simulink model "non_linear_final"  (R2021b)
settings: Solver=ode23  StartTime=0.0  StopTime=30  RelTol=1e-3
values from neighbouring files (symbols this model uses):
  A_ratio = 1.41    [non_linear_param_final.m]
  Annulus_area = Bore_area-Rod_area    (= 2356.19449019)    [non_linear_param_final.m]
  beta_F = 12000e5    (= 1200000000)    [non_linear_param_final.m]
  Bore_area = pi/4*Bore_diameter^2    (= 3318.30724035)    [non_linear_param_final.m]
  cyl_c_end = 9e8    (= 900000000)    [non_linear_param_final.m]
  cyl_c_visc = 1500    [non_linear_param_final.m]
  cyl_CH_stat = 1/50    (= 0.02)    [non_linear_param_final.m]
  cyl_F_coul = 75    [non_linear_param_final.m]
  cyl_FH_stat = 500    [non_linear_param_final.m]
  cyl_gamma = 250    [non_linear_param_final.m]
  cyl_k_end = 6e9    (= 6000000000)    [non_linear_param_final.m]
  DB = -0.114    [non_linear_param_final.m]
  dr_dcv = 0.7    [non_linear_param_final.m]
  Eff_Bulk_On = 1    [non_linear_param_final.m]
  eta_air = 0.7e-2    (= 0.007)    [non_linear_param_final.m]
  k_cv = 15    [non_linear_param_final.m]
  k_DCV_PA = 7.9    [non_linear_param_final.m]
  k_DCV_PB = 7.78    [non_linear_param_final.m]
  k_DCV_T = 7.9    [non_linear_param_final.m]
  k_ocv = 15    [non_linear_param_final.m]
  K_oil = 1.5e4*0+12000    (= 12000)    [non_linear_param_final.m]
  k_tanh = 0.005    [non_linear_param_final.m]
  kappa_air = 1.4    [non_linear_param_final.m]
  LargeValveOpening = 1    [non_linear_param_final.m]
  mu_ocv = 3    [non_linear_param_final.m]
  OL_pa = -0.114    [non_linear_param_final.m]
  OL_pb = 0.114    [non_linear_param_final.m]
  p0_A = 62.79    [non_linear_param_final.m]
  p0_A1 = 1.08    [non_linear_param_final.m]
  p0_B = 1.213    [non_linear_param_final.m]
  p_cv_fo = 1    [non_linear_param_final.m]
  p_cv_set = 2.8    [non_linear_param_final.m]
  p_ocv_fo = 350    [non_linear_param_final.m]
  p_ocv_set = 150    [non_linear_param_final.m]
  p_pc_set = 7    [non_linear_param_final.m]
  p_R = 1.1    [non_linear_param_final.m]
  p_S = 180    [non_linear_param_final.m]
  patm = 1.013e5    (= 101300)    [non_linear_param_final.m]
  Stroke = 500    [non_linear_param_final.m]
  t0 = 1    [non_linear_param_final.m]
  tau_ocv = 0.0889*0+0.055    (= 0.055)    [non_linear_param_final.m]
  tau_pc = 0.0064*0+0.009    (= 0.009)    [non_linear_param_final.m]
  tStepDown = 4.7*0+4.6    (= 4.6)    [non_linear_param_final.m]
  tStepUp = 6.2    [non_linear_param_final.m]
  tWait = 8.0    (= 8)    [non_linear_param_final.m]
  UL_at = -0.05    [non_linear_param_final.m]
  UL_bt = 0.05    [non_linear_param_final.m]
  Up = 1    [non_linear_param_final.m]
  uS_sp = -0.55    [non_linear_param_final.m]
  V_CH_A = 1.0e-03    (= 0.001)    [non_linear_param_final.m]
  V_CH_A1 = 0.1e-03    (= 0.0001)    [non_linear_param_final.m]
  V_CH_B = 1.25e-03    (= 0.00125)    [non_linear_param_final.m]
  v_cyl_0 = 0    [non_linear_param_final.m]
  wn_dcv = 30    [non_linear_param_final.m]
  x_cyl_0 = 0.45012    [non_linear_param_final.m]
undefined symbols (not defined in the file or in its folder): sigbuilder_block, uS0
libraries: eeGeneralControl x3
(part 1 of 2)
settings Model: Creator=Daniel Hagen  LastModifiedBy=danielh
settings SolverCC: ZeroCrossAlgorithm=Adaptive  AlgebraicLoopSolver=TrustRegion
settings DataIOCC: ReturnWorkspaceOutputs=off
settings DebuggingCC: IgnoredZcDiagnostic=none  SFMachineParentedDataDiag=warning  ArithmeticOperatorsInVariantConditions=warning
settings SFSimCC: CustomCodeUndefinedFunction=UseInterfaceOnly

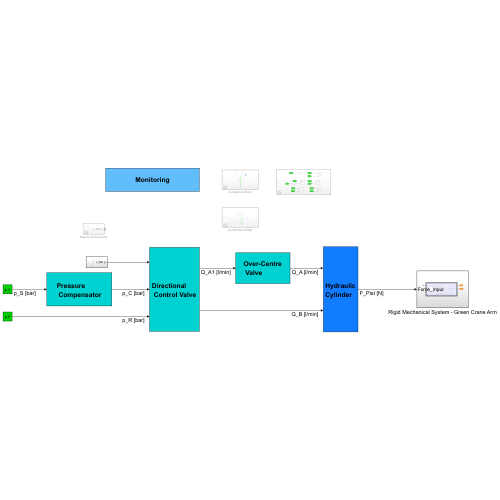
[thumbnail: Simulink's stored preview of the top-level diagram]
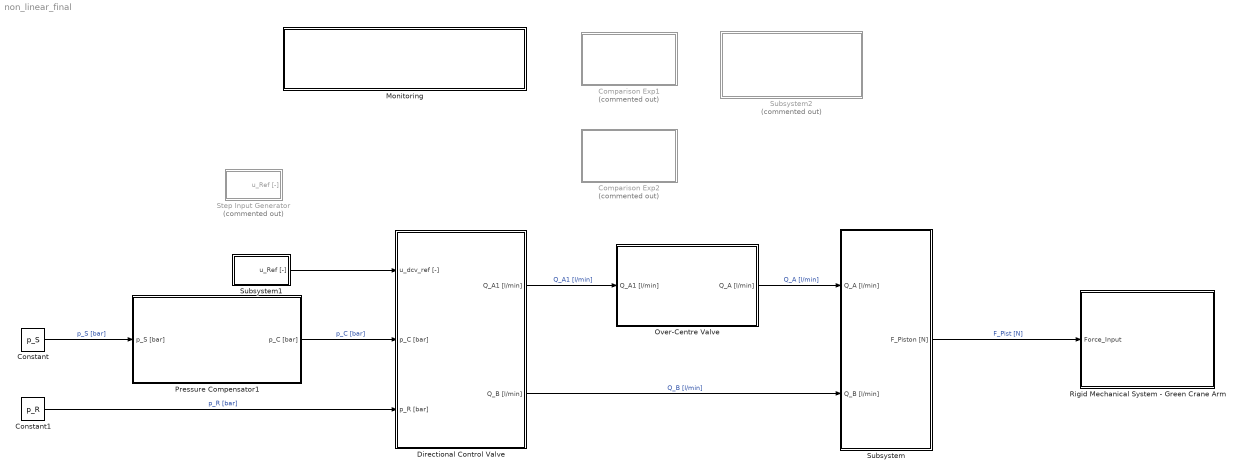
== system non_linear_final ==
  Comparison Exp1 : Subsystem  Commented=on
  Comparison Exp2 : Subsystem  Commented=on
  Constant : Constant  Value=p_S
  Constant1 : Constant  Value=p_R
  Directional Control Valve : Subsystem
      mask Display: disp('{\bf\fontsize{22}Directional\newline Control Valve}', 'texmode','on');
  Monitoring : Subsystem
      mask Display: disp('{\bf\fontsize{22}Monitoring}', 'texmode','on');
  Over-Centre Valve : Subsystem
      mask Display: disp('{\bf\fontsize{22}Over-Centre\newline Valve}', 'texmode','on');
  Pressure Compensator1 : Subsystem
      mask Display: disp('{\bf\fontsize{22}Pressure\newline Compensator}', 'texmode','on');
  Rigid Mechanical System - Green Crane Arm : Subsystem  VariantControl=Rigid_model
  Step Input Generator : Subsystem  Commented=on
  Subsystem : Subsystem
      mask Display: disp('{\bf\fontsize{22} Hydraulic \newline Cylinder}', 'texmode','on');
  Subsystem1 : Subsystem
  Subsystem2 : Subsystem  Commented=on
  wires:
  Directional Control Valve/1(Q_A1 [l/min]) -> Over-Centre Valve/1(Q_A1 [l/min])  "Q_A1 [l/min]"
  Subsystem/1(F_Piston [N]) -> Rigid Mechanical System - Green Crane Arm/1(Force_Input)  "F_Pist [N]"
  Pressure Compensator1/1(p_C [bar]) -> Directional Control Valve/2(p_C [bar])  "p_C [bar]"
  Constant/1 -> Pressure Compensator1/1(p_S [bar])  "p_S [bar]"
  Over-Centre Valve/1(Q_A [l/min]) -> Subsystem/1(Q_A [l/min])  "Q_A [l/min]"
  Constant1/1 -> Directional Control Valve/3(p_R [bar])  "p_R [bar]"
  Directional Control Valve/2(Q_B [l/min]) -> Subsystem/2(Q_B [l/min])  "Q_B [l/min]"
  Subsystem1/1(u_Ref [-]) -> Directional Control Valve/1(u_dcv_ref [-])

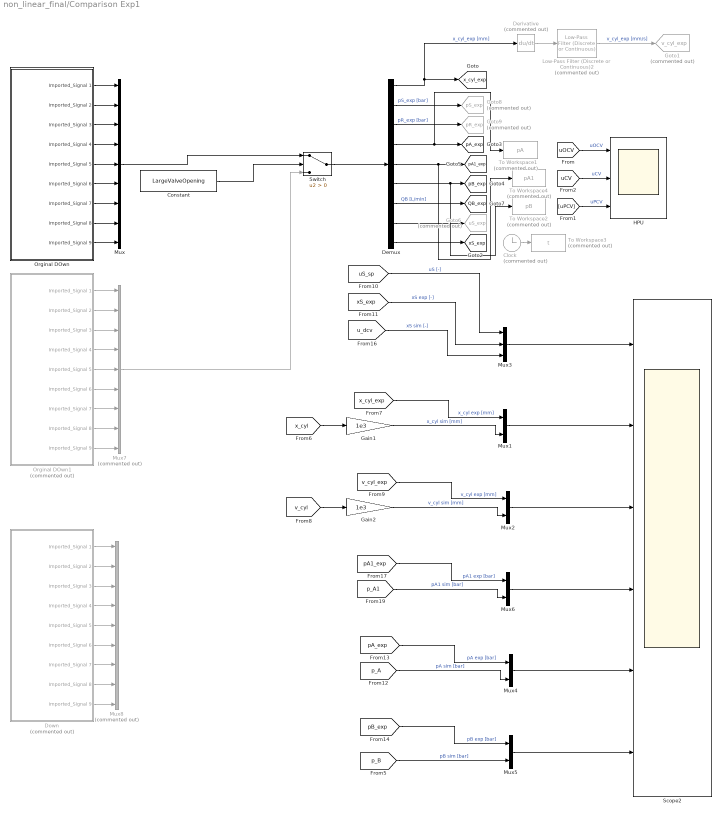
== system non_linear_final/Comparison Exp1 ==
  Clock : Clock  Commented=on
  Constant : Constant  Value=LargeValveOpening
  Demux : Demux  Outputs=9
  Derivative : Derivative  Commented=on
  Down : Subsystem  Tag=STV Subsys  Commented=on  LoadFcn=sigbuilder_block('load');  StartFcn=sigbuilder_block('start');  StopFcn=sigbuilder_block('stop');  ClipboardFcn=sigbuilder_block('clipboard');  mask(Sigbuilder block){tuvar=[0:10]}
      mask help: Simulink:masks:SigbuilderBlock_MD
      mask init:
      | if ~strcmp(get_param(bdroot(gcbh),'SimulationStatus'),'stopped') tuvar = sigbuilder_block('maskInit'); end
      settings: CopyFcn=sigbuilder_block('copy');  DeleteFcn=sigbuilder_block('delete');  ModelCloseFcn=sigbuilder_block('modelClose');  PreSaveFcn=sigbuilder_block('preSave');  PauseFcn=sigbuilder_block('pause');  ContinueFcn=sigbuilder_block('continue');  NameChangeFcn=sigbuilder_block('namechange');  OpenFcn=sigbuilder_block('open',[41.25 0 1392.75 801.75 ]);  CloseFcn=sigbuilder_block('close');
      mask Display: plot(0, 0, 100, 100,[2, 2, 32, 32, 2], [68, 8, 8, 68, 68],[32, 2], [38, 38], [32, 19, 2],[53, 60, 44], [32, 17, 17, 2],[16, 16, 31, 31]);txt = getActiveGroup(gcbh);text(2, 100, txt,'verticalAlignment', 'top');
  Gain1 : Gain  Gain=1e3
  Gain2 : Gain  Gain=1e3
  Goto1 : Goto  Commented=on  GotoTag=v_cyl_exp  TagVisibility=global
  Goto6 : Goto  Commented=on  GotoTag=uS_exp  TagVisibility=global
  Goto8 : Goto  Commented=on  GotoTag=pS_exp  TagVisibility=global
  Goto9 : Goto  Commented=on  GotoTag=pR_exp  TagVisibility=global
  HPU : Scope  NumInputPorts=3
  Low-Pass Filter (Discrete or Continuous)2 : eeGeneralControl/Low-Pass Filter (Discrete or Continuous)  Commented=on  K=1  T=0.05  B=150  A=-150  init_option=Inherited from block input  x0=0  Ts=0.001
  Mux : Mux  Inputs=9
  Mux{1..2,4..6} (5 alike) : Mux  Inputs=2
  Mux3 : Mux  Inputs=3
  Mux7 : Mux  Commented=on  Inputs=9
  Mux8 : Mux  Commented=on  Inputs=9
  Orginal DOwn : Subsystem  Tag=STV Subsys  LoadFcn=sigbuilder_block('load');  StartFcn=sigbuilder_block('start');  StopFcn=sigbuilder_block('stop');  ClipboardFcn=sigbuilder_block('clipboard');  mask(Sigbuilder block){tuvar=[0:10]}
      mask help: Simulink:masks:SigbuilderBlock_MD
      mask init:
      | if ~strcmp(get_param(bdroot(gcbh),'SimulationStatus'),'stopped') tuvar = sigbuilder_block('maskInit'); end
      settings: CopyFcn=sigbuilder_block('copy');  DeleteFcn=sigbuilder_block('delete');  ModelCloseFcn=sigbuilder_block('modelClose');  PreSaveFcn=sigbuilder_block('preSave');  PauseFcn=sigbuilder_block('pause');  ContinueFcn=sigbuilder_block('continue');  NameChangeFcn=sigbuilder_block('namechange');  OpenFcn=sigbuilder_block('open',[437.6 199.2 550.4 400 ]);  CloseFcn=sigbuilder_block('close');
      mask Display: plot(0, 0, 100, 100,[2, 2, 32, 32, 2], [68, 8, 8, 68, 68],[32, 2], [38, 38], [32, 19, 2],[53, 60, 44], [32, 17, 17, 2],[16, 16, 31, 31]);txt = getActiveGroup(gcbh);text(2, 100, txt,'verticalAlignment', 'top');
  Orginal DOwn1 : Subsystem  Tag=STV Subsys  Commented=on  LoadFcn=sigbuilder_block('load');  StartFcn=sigbuilder_block('start');  StopFcn=sigbuilder_block('stop');  ClipboardFcn=sigbuilder_block('clipboard');  mask(Sigbuilder block){tuvar=[0:10]}
      mask help: Simulink:masks:SigbuilderBlock_MD
      mask init:
      | if ~strcmp(get_param(bdroot(gcbh),'SimulationStatus'),'stopped') tuvar = sigbuilder_block('maskInit'); end
      settings: CopyFcn=sigbuilder_block('copy');  DeleteFcn=sigbuilder_block('delete');  ModelCloseFcn=sigbuilder_block('modelClose');  PreSaveFcn=sigbuilder_block('preSave');  PauseFcn=sigbuilder_block('pause');  ContinueFcn=sigbuilder_block('continue');  NameChangeFcn=sigbuilder_block('namechange');  OpenFcn=sigbuilder_block('open',[47.25 0 1392.75 842.25 ]);  CloseFcn=sigbuilder_block('close');
      mask Display: plot(0, 0, 100, 100,[2, 2, 32, 32, 2], [68, 8, 8, 68, 68],[32, 2], [38, 38], [32, 19, 2],[53, 60, 44], [32, 17, 17, 2],[16, 16, 31, 31]);txt = getActiveGroup(gcbh);text(2, 100, txt,'verticalAlignment', 'top');
  Scope2 : Scope  NumInputPorts=6
  Switch : Switch  Criteria=u2 > Threshold
  To Workspace1 : ToWorkspace  Commented=on  VariableName=pA  SampleTime=0.001
      settings: Save2DSignal=2-D array (concatenate along first dimension)
  To Workspace2 : ToWorkspace  Commented=on  VariableName=pB  SampleTime=0.001
      settings: Save2DSignal=2-D array (concatenate along first dimension)
  To Workspace3 : ToWorkspace  Commented=on  VariableName=t  SampleTime=0.001
      settings: Save2DSignal=2-D array (concatenate along first dimension)
  To Workspace4 : ToWorkspace  Commented=on  VariableName=pA1  SampleTime=0.001
      settings: Save2DSignal=2-D array (concatenate along first dimension)
  tag scope: QB_exp=global, pA1_exp=global, pA_exp=global, pB_exp=global, xS_exp=global, x_cyl_exp=global
  wires:
  Demux/9 -> [xS_exp]
  Demux/1 -> [x_cyl_exp], Derivative/1  "x_cyl_exp [mm]"
  Demux/5 -> To Workspace4/1, [pA1_exp]
  Low-Pass Filter (Discrete or Continuous)2/1 -> Goto1/1  "v_cyl_exp [mm/s]"
  Demux/6 -> To Workspace2/1, [pB_exp]
  Derivative/1 -> Low-Pass Filter (Discrete or Continuous)2/1
  Demux/4 -> To Workspace1/1, [pA_exp]
  Demux/7 -> [QB_exp]  "QB [L/min]"
  Demux/8 -> Goto6/1
  Demux/3 -> Goto9/1  "pR_exp [bar]"
  Demux/2 -> Goto8/1  "pS_exp [bar]"
  Orginal DOwn/4(Imported_Signal 4) -> Mux/4
  Orginal DOwn/5(Imported_Signal 5) -> Mux/5
  Orginal DOwn/6(Imported_Signal 6) -> Mux/6
  Orginal DOwn/7(Imported_Signal 7) -> Mux/7
  Orginal DOwn/8(Imported_Signal 8) -> Mux/8
  Orginal DOwn/9(Imported_Signal 9) -> Mux/9
  Orginal DOwn/3(Imported_Signal 3) -> Mux/3
  Orginal DOwn/1(Imported_Signal 1) -> Mux/1
  Orginal DOwn/2(Imported_Signal 2) -> Mux/2
  Orginal DOwn1/3(Imported_Signal 3) -> Mux7/3
  Orginal DOwn1/1(Imported_Signal 1) -> Mux7/1
  Orginal DOwn1/5(Imported_Signal 5) -> Mux7/5
  Orginal DOwn1/7(Imported_Signal 7) -> Mux7/7
  Orginal DOwn1/6(Imported_Signal 6) -> Mux7/6
  Orginal DOwn1/2(Imported_Signal 2) -> Mux7/2
  Orginal DOwn1/9(Imported_Signal 9) -> Mux7/9
  Orginal DOwn1/4(Imported_Signal 4) -> Mux7/4
  Mux7/1 -> Switch/3
  Orginal DOwn1/8(Imported_Signal 8) -> Mux7/8
  Mux/1 -> Switch/1
  Constant/1 -> Switch/2
  [x_cyl_exp] -> Mux1/1  "x_cyl exp [mm]"
  [x_cyl] -> Gain1/1
  [p_A] -> Mux4/2  "pA sim [bar]"
  [v_cyl] -> Gain2/1
  Mux4/1 -> Scope2/5
  Mux2/1 -> Scope2/3
  [xS_exp] -> Mux3/2  "xS exp [-]"
  [pA_exp] -> Mux4/1  "pA exp [bar]"
  Gain1/1 -> Mux1/2  "x_cyl sim [mm]"
  Gain2/1 -> Mux2/2  "v_cyl sim [mm]"
  [p_A1] -> Mux6/2  "pA1 sim [bar]"
  [pB_exp] -> Mux5/1  "pB exp [bar]"
  [u_dcv] -> Mux3/3  "xS sim [-]"
  Mux3/1 -> Scope2/1
  [uS_sp] -> Mux3/1  "uS [-]"
  [p_B] -> Mux5/2  "pB sim [bar]"
  Mux6/1 -> Scope2/4
  Mux1/1 -> Scope2/2
  [v_cyl_exp] -> Mux2/1  "v_cyl exp [mm]"
  [pA1_exp] -> Mux6/1  "pA1 exp [bar]"
  Mux5/1 -> Scope2/6
  Down/2(Imported_Signal 2) -> Mux8/2
  Down/3(Imported_Signal 3) -> Mux8/3
  Down/4(Imported_Signal 4) -> Mux8/4
  Down/9(Imported_Signal 9) -> Mux8/9
  Down/1(Imported_Signal 1) -> Mux8/1
  Down/7(Imported_Signal 7) -> Mux8/7
  Down/5(Imported_Signal 5) -> Mux8/5
  Down/8(Imported_Signal 8) -> Mux8/8
  Down/6(Imported_Signal 6) -> Mux8/6
  Switch/1 -> Demux/1
  [uOCV] -> HPU/1  "uOCV"
  [uPCV] -> HPU/3  "uPCV"
  [uCV] -> HPU/2  "uCV"
  Clock/1 -> To Workspace3/1

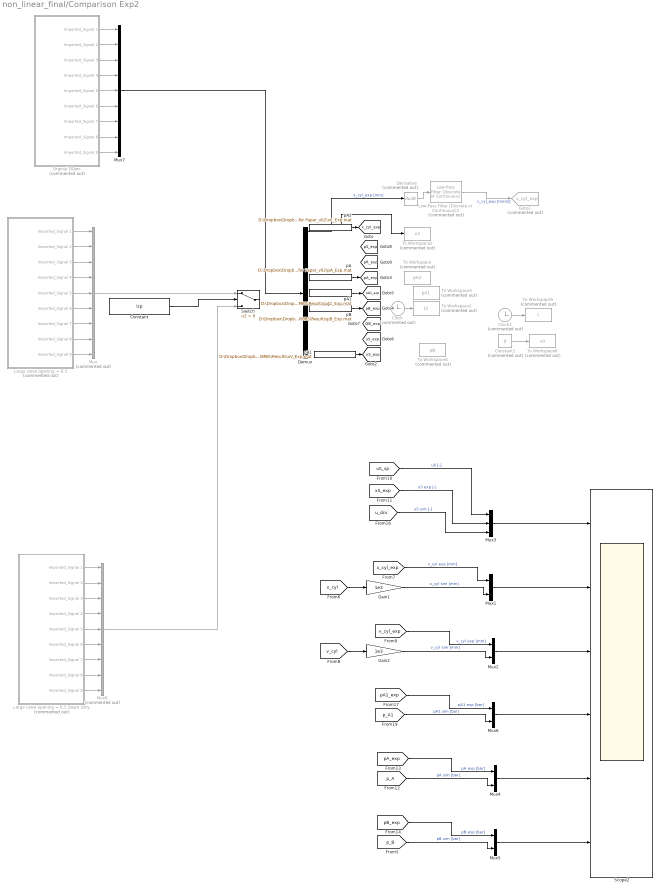
== system non_linear_final/Comparison Exp2 ==
  Clock : Clock  Commented=on
  Clock1 : Clock  Commented=on
  Constant : Constant  Value=Up
  Constant1 : Constant  Commented=on  Value=0
  Demux : Demux  Outputs=9
  Derivative : Derivative  Commented=on
  Gain1 : Gain  Gain=1e3
  Gain2 : Gain  Gain=1e3
  Goto1 : Goto  Commented=on  GotoTag=v_cyl_exp  TagVisibility=global
  Large valve opening = 0.5 : Subsystem  Tag=STV Subsys  Commented=on  LoadFcn=sigbuilder_block('load');  StartFcn=sigbuilder_block('start');  StopFcn=sigbuilder_block('stop');  ClipboardFcn=sigbuilder_block('clipboard');  mask(Sigbuilder block){tuvar=[0:10]}
      mask help: Simulink:masks:SigbuilderBlock_MD
      mask init:
      | if ~strcmp(get_param(bdroot(gcbh),'SimulationStatus'),'stopped') tuvar = sigbuilder_block('maskInit'); end
      settings: CopyFcn=sigbuilder_block('copy');  DeleteFcn=sigbuilder_block('delete');  ModelCloseFcn=sigbuilder_block('modelClose');  PreSaveFcn=sigbuilder_block('preSave');  PauseFcn=sigbuilder_block('pause');  ContinueFcn=sigbuilder_block('continue');  NameChangeFcn=sigbuilder_block('namechange');  OpenFcn=sigbuilder_block('open',[41.25 0 1392.75 801.75 ]);  CloseFcn=sigbuilder_block('close');
      mask Display: plot(0, 0, 100, 100,[2, 2, 32, 32, 2], [68, 8, 8, 68, 68],[32, 2], [38, 38], [32, 19, 2],[53, 60, 44], [32, 17, 17, 2],[16, 16, 31, 31]);txt = getActiveGroup(gcbh);text(2, 100, txt,'verticalAlignment', 'top');
  Large valve opening = 0.5 Down Only : Subsystem  Tag=STV Subsys  Commented=on  LoadFcn=sigbuilder_block('load');  StartFcn=sigbuilder_block('start');  StopFcn=sigbuilder_block('stop');  ClipboardFcn=sigbuilder_block('clipboard');  mask(Sigbuilder block){tuvar=[0:10]}
      mask help: Simulink:masks:SigbuilderBlock_MD
      mask init:
      | if ~strcmp(get_param(bdroot(gcbh),'SimulationStatus'),'stopped') tuvar = sigbuilder_block('maskInit'); end
      settings: CopyFcn=sigbuilder_block('copy');  DeleteFcn=sigbuilder_block('delete');  ModelCloseFcn=sigbuilder_block('modelClose');  PreSaveFcn=sigbuilder_block('preSave');  PauseFcn=sigbuilder_block('pause');  ContinueFcn=sigbuilder_block('continue');  NameChangeFcn=sigbuilder_block('namechange');  OpenFcn=sigbuilder_block('open',[41.25 0 1392.75 801.75 ]);  CloseFcn=sigbuilder_block('close');
      mask Display: plot(0, 0, 100, 100,[2, 2, 32, 32, 2], [68, 8, 8, 68, 68],[32, 2], [38, 38], [32, 19, 2],[53, 60, 44], [32, 17, 17, 2],[16, 16, 31, 31]);txt = getActiveGroup(gcbh);text(2, 100, txt,'verticalAlignment', 'top');
  Low-Pass Filter (Discrete or Continuous)2 : eeGeneralControl/Low-Pass Filter (Discrete or Continuous)  Commented=on  K=1  T=0.05  B=150  A=-150  init_option=Inherited from block input  x0=0  Ts=0.001
  Mux : Mux  Commented=on  Inputs=9
  Mux{1..2,4..6} (5 alike) : Mux  Inputs=2
  Mux3 : Mux  Inputs=3
  Mux7 : Mux  Inputs=9
  Mux8 : Mux  Commented=on  Inputs=9
  Orginal DOwn : Subsystem  Tag=STV Subsys  Commented=on  LoadFcn=sigbuilder_block('load');  StartFcn=sigbuilder_block('start');  StopFcn=sigbuilder_block('stop');  ClipboardFcn=sigbuilder_block('clipboard');  mask(Sigbuilder block){tuvar=[0:10]}
      mask help: Simulink:masks:SigbuilderBlock_MD
      mask init:
      | if ~strcmp(get_param(bdroot(gcbh),'SimulationStatus'),'stopped') tuvar = sigbuilder_block('maskInit'); end
      settings: CopyFcn=sigbuilder_block('copy');  DeleteFcn=sigbuilder_block('delete');  ModelCloseFcn=sigbuilder_block('modelClose');  PreSaveFcn=sigbuilder_block('preSave');  PauseFcn=sigbuilder_block('pause');  ContinueFcn=sigbuilder_block('continue');  NameChangeFcn=sigbuilder_block('namechange');  OpenFcn=sigbuilder_block('open',[437.6 199.2 550.4 400 ]);  CloseFcn=sigbuilder_block('close');
      mask Display: plot(0, 0, 100, 100,[2, 2, 32, 32, 2], [68, 8, 8, 68, 68],[32, 2], [38, 38], [32, 19, 2],[53, 60, 44], [32, 17, 17, 2],[16, 16, 31, 31]);txt = getActiveGroup(gcbh);text(2, 100, txt,'verticalAlignment', 'top');
  Scope2 : Scope  NumInputPorts=6
  Switch : Switch  Criteria=u2 > Threshold
  To Workspace : ToWorkspace  Commented=on  VariableName=pA2  SampleTime=0.001
      settings: Save2DSignal=2-D array (concatenate along first dimension)
  To Workspace1 : ToWorkspace  Commented=on  VariableName=t2  SampleTime=0.001
      settings: Save2DSignal=2-D array (concatenate along first dimension)
  To Workspace2 : ToWorkspace  Commented=on  VariableName=x2  SampleTime=0.001
      settings: Save2DSignal=2-D array (concatenate along first dimension)
  To Workspace3 : ToWorkspace  Commented=on  VariableName=pA1  SampleTime=0.001
      settings: Save2DSignal=2-D array (concatenate along first dimension)
  To Workspace4 : ToWorkspace  Commented=on  VariableName=pB  SampleTime=0.001
      settings: Save2DSignal=2-D array (concatenate along first dimension)
  To Workspace5 : ToWorkspace  Commented=on  VariableName=t  SampleTime=0.001
      settings: Save2DSignal=2-D array (concatenate along first dimension)
  To Workspace6 : ToWorkspace  Commented=on  VariableName=v0  SampleTime=0.001
      settings: Save2DSignal=2-D array (concatenate along first dimension)
  pA : FromFile  FileName=D:\Dropbox\Dropbox\PhD\Models\LSS model\Comparison_Paper_Model\Non Linear Model\Results for Paper_v02\pA_Exp.mat  SampleTime=0
  pA1 : FromFile  FileName=D:\Dropbox\Dropbox\PhD\Models\LSS model\Comparison_Paper_Model\Non Linear Model\MBS\Results\pA1_Exp.mat  SampleTime=0
  pA2 : FromFile  FileName=D:\Dropbox\Dropbox\PhD\Models\LSS model\Comparison_Paper_Model\Non Linear Model\Results for Paper_v02\xC_Exp.mat  SampleTime=0
  pB : FromFile  FileName=D:\Dropbox\Dropbox\PhD\Models\LSS model\Comparison_Paper_Model\Non Linear Model\MBS\Results\pB_Exp.mat  SampleTime=0
  pB1 : FromFile  FileName=D:\Dropbox\Dropbox\PhD\Models\LSS model\Comparison_Paper_Model\Non Linear Model\MBS\Results\xV_Exp.mat  SampleTime=0
  tag scope: QB_exp=global, pA1_exp=global, pA_exp=global, pB_exp=global, pR_exp=global, pS_exp=global, uS_exp=global, xS_exp=global, x_cyl_exp=global
  wires:
  Demux/1 -> To Workspace2/1, Derivative/1  "x_cyl_exp [mm]"
  Low-Pass Filter (Discrete or Continuous)2/1 -> Goto1/1  "v_cyl_exp [mm/s]"
  Derivative/1 -> Low-Pass Filter (Discrete or Continuous)2/1
  Large valve opening = 0.5/4(Imported_Signal 4) -> Mux/4
  Large valve opening = 0.5/5(Imported_Signal 5) -> Mux/5
  Large valve opening = 0.5/6(Imported_Signal 6) -> Mux/6
  Large valve opening = 0.5/7(Imported_Signal 7) -> Mux/7
  Large valve opening = 0.5/8(Imported_Signal 8) -> Mux/8
  Large valve opening = 0.5/9(Imported_Signal 9) -> Mux/9
  Large valve opening = 0.5/3(Imported_Signal 3) -> Mux/3
  Large valve opening = 0.5/1(Imported_Signal 1) -> Mux/1
  Large valve opening = 0.5/2(Imported_Signal 2) -> Mux/2
  Mux/1 -> Switch/1
  Constant/1 -> Switch/2
  [x_cyl] -> Gain1/1
  [p_A] -> Mux4/2  "pA sim [bar]"
  [v_cyl] -> Gain2/1
  Mux4/1 -> Scope2/5
  Mux2/1 -> Scope2/3
  [xS_exp] -> Mux3/2  "xS exp [-]"
  [pA_exp] -> Mux4/1  "pA exp [bar]"
  Gain1/1 -> Mux1/2  "x_cyl sim [mm]"
  Gain2/1 -> Mux2/2  "v_cyl sim [mm]"
  [p_A1] -> Mux6/2  "pA1 sim [bar]"
  [pB_exp] -> Mux5/1  "pB exp [bar]"
  [u_dcv] -> Mux3/3  "xS sim [-]"
  Mux3/1 -> Scope2/1
  [uS_sp] -> Mux3/1  "uS [-]"
  [p_B] -> Mux5/2  "pB sim [bar]"
  Mux6/1 -> Scope2/4
  Mux1/1 -> Scope2/2
  [v_cyl_exp] -> Mux2/1  "v_cyl exp [mm]"
  [pA1_exp] -> Mux6/1  "pA1 exp [bar]"
  Mux5/1 -> Scope2/6
  Large valve opening = 0.5 Down Only/4(Imported_Signal 4) -> Mux8/4
  Large valve opening = 0.5 Down Only/8(Imported_Signal 8) -> Mux8/8
  Large valve opening = 0.5 Down Only/1(Imported_Signal 1) -> Mux8/1
  Large valve opening = 0.5 Down Only/2(Imported_Signal 2) -> Mux8/2
  Large valve opening = 0.5 Down Only/6(Imported_Signal 6) -> Mux8/6
  Large valve opening = 0.5 Down Only/9(Imported_Signal 9) -> Mux8/9
  Large valve opening = 0.5 Down Only/3(Imported_Signal 3) -> Mux8/3
  Large valve opening = 0.5 Down Only/5(Imported_Signal 5) -> Mux8/5
  Large valve opening = 0.5 Down Only/7(Imported_Signal 7) -> Mux8/7
  Mux8/1 -> Switch/3
  Orginal DOwn/1(Imported_Signal 1) -> Mux7/1
  Orginal DOwn/8(Imported_Signal 8) -> Mux7/8
  Orginal DOwn/2(Imported_Signal 2) -> Mux7/2
  Orginal DOwn/9(Imported_Signal 9) -> Mux7/9
  Orginal DOwn/6(Imported_Signal 6) -> Mux7/6
  Orginal DOwn/5(Imported_Signal 5) -> Mux7/5
  Orginal DOwn/3(Imported_Signal 3) -> Mux7/3
  Orginal DOwn/7(Imported_Signal 7) -> Mux7/7
  Orginal DOwn/4(Imported_Signal 4) -> Mux7/4
  Clock/1 -> To Workspace1/1
  Mux7/1 -> Demux/1
  Clock1/1 -> To Workspace5/1
  Constant1/1 -> To Workspace6/1
  [x_cyl_exp] -> Mux1/1  "x_cyl exp [mm]"
  pA/1 -> [pA_exp]
  pA1/1 -> [pA1_exp]
  pB/1 -> [pB_exp]
  pB1/1 -> [xS_exp]
  pA2/1 -> [x_cyl_exp]

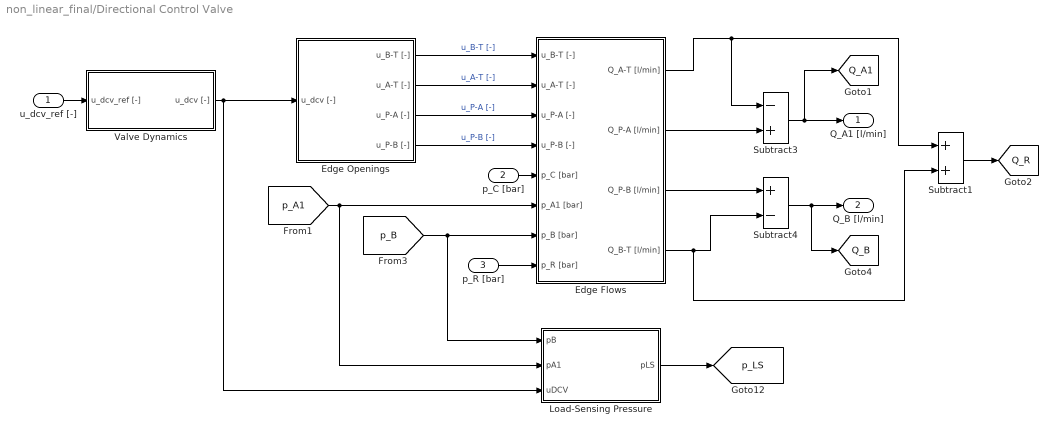
== system non_linear_final/Directional Control Valve ==
  u_dcv_ref [-] : Inport
  p_C [bar] : Inport  Port=2
  p_R [bar] : Inport  Port=3
  Edge Flows : Subsystem
  Edge Openings : Subsystem
  Load-Sensing Pressure : Subsystem
  Subtract1 : Sum
  Subtract3 : Sum  Inputs=-+
  Subtract4 : Sum  Inputs=+-
  Valve Dynamics : Subsystem
  Q_A1 [l/min] : Outport  VectorParamsAs1DForOutWhenUnconnected=off
  Q_B [l/min] : Outport  Port=2  VectorParamsAs1DForOutWhenUnconnected=off
  tag scope: Q_A1=global, Q_B=global, Q_R=global, p_LS=global
  wires:
  Valve Dynamics/1(u_dcv [-]) -> Load-Sensing Pressure/3(uDCV), Edge Openings/1(u_dcv [-])
  Edge Flows/2(Q_P-A [l/min]) -> Subtract3/2
  u_dcv_ref [-]/1 -> Valve Dynamics/1(u_dcv_ref [-])
  p_R [bar]/1 -> Edge Flows/8(p_R [bar])
  [p_A1] -> Load-Sensing Pressure/2(pA1), Edge Flows/6(p_A1 [bar])
  Subtract3/1 -> [Q_A1], Q_A1 [l/min]/1
  Subtract4/1 -> [Q_B], Q_B [l/min]/1
  [p_B] -> Load-Sensing Pressure/1(pB), Edge Flows/7(p_B [bar])
  p_C [bar]/1 -> Edge Flows/5(p_C [bar])
  Edge Flows/4(Q_B-T [l/min]) -> Subtract1/2, Subtract4/2
  Subtract1/1 -> [Q_R]
  Edge Openings/2(u_A-T [-]) -> Edge Flows/2(u_A-T [-])  "u_A-T [-]"
  Edge Openings/4(u_P-B [-]) -> Edge Flows/4(u_P-B [-])  "u_P-B [-]"
  Edge Openings/1(u_B-T [-]) -> Edge Flows/1(u_B-T [-])  "u_B-T [-]"
  Edge Openings/3(u_P-A [-]) -> Edge Flows/3(u_P-A [-])  "u_P-A [-]"
  Edge Flows/3(Q_P-B [l/min]) -> Subtract4/1
  Edge Flows/1(Q_A-T [l/min]) -> Subtract3/1, Subtract1/1
  Load-Sensing Pressure/1(pLS) -> [p_LS]
